simulink model "v01_aa_rii_16r4t11f_ver122sa"  (Simulink 7.6)
settings: Solver=ode45  StartTime=0.0  StopTime=128000  RelTol=1e-3
undefined symbols (not defined in the file or in its folder): sfp
libraries: xbsIndex_r4 x27287, monroe_library x154, xbsReplacements_r4 x78, casper_library_misc x33, simulink x32, dspsigops x32, xps_library x24
(part 33 of 178)

== system v01_aa_rii_16r4t11f_ver122sa/pfb_fir_real1/coeff_gen_6_9/cram_upper ==
  in0 : Inport
  in1 : Inport  Port=2
  in2 : Inport  Port=3
  in3 : Inport  Port=4
  concat : xbsIndex_r4/Concat  num_inputs=4  dbl_ovrd=off  block_type=concat
  reinterpret_{1..4} (4 alike) : xbsIndex_r4/Reinterpret  force_arith_type=on  arith_type=Unsigned  force_bin_pt=on  bin_pt=0  block_type=reinterpret
  out : Outport
  wires:
  concat/1 -> out/1
  reinterpret_4/1 -> concat/4
  reinterpret_3/1 -> concat/3
  reinterpret_2/1 -> concat/2
  reinterpret_1/1 -> concat/1
  in3/1 -> reinterpret_4/1
  in2/1 -> reinterpret_3/1
  in1/1 -> reinterpret_2/1
  in0/1 -> reinterpret_1/1

== system v01_aa_rii_16r4t11f_ver122sa/pfb_fir_real1/coeff_gen_7_8/cram_lower ==
  in0 : Inport
  in1 : Inport  Port=2
  in2 : Inport  Port=3
  in3 : Inport  Port=4
  concat : xbsIndex_r4/Concat  num_inputs=4  dbl_ovrd=off  block_type=concat
  reinterpret_{1..4} (4 alike) : xbsIndex_r4/Reinterpret  force_arith_type=on  arith_type=Unsigned  force_bin_pt=on  bin_pt=0  block_type=reinterpret
  out : Outport
  wires:
  concat/1 -> out/1
  reinterpret_4/1 -> concat/4
  reinterpret_3/1 -> concat/3
  reinterpret_2/1 -> concat/2
  reinterpret_1/1 -> concat/1
  in3/1 -> reinterpret_4/1
  in2/1 -> reinterpret_3/1
  in1/1 -> reinterpret_2/1
  in0/1 -> reinterpret_1/1

== system v01_aa_rii_16r4t11f_ver122sa/pfb_fir_real1/coeff_gen_7_8/cram_upper ==
  in0 : Inport
  in1 : Inport  Port=2
  in2 : Inport  Port=3
  in3 : Inport  Port=4
  concat : xbsIndex_r4/Concat  num_inputs=4  dbl_ovrd=off  block_type=concat
  reinterpret_{1..4} (4 alike) : xbsIndex_r4/Reinterpret  force_arith_type=on  arith_type=Unsigned  force_bin_pt=on  bin_pt=0  block_type=reinterpret
  out : Outport
  wires:
  concat/1 -> out/1
  reinterpret_4/1 -> concat/4
  reinterpret_3/1 -> concat/3
  reinterpret_2/1 -> concat/2
  reinterpret_1/1 -> concat/1
  in3/1 -> reinterpret_4/1
  in2/1 -> reinterpret_3/1
  in1/1 -> reinterpret_2/1
  in0/1 -> reinterpret_1/1

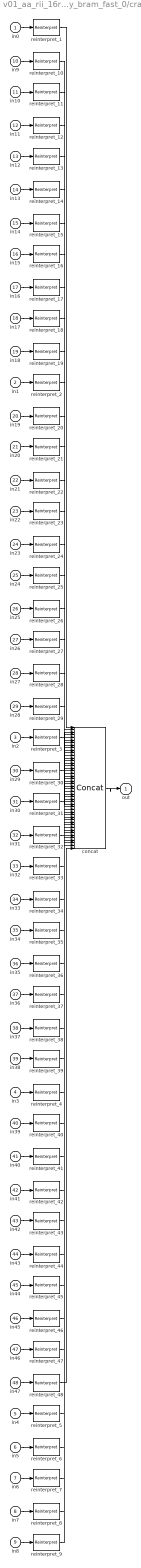
== system v01_aa_rii_16r4t11f_ver122sa/pfb_fir_real1/multi_delay_bram_fast_0/cram ==
  in0 : Inport
  in1 : Inport  Port=2
  in2 : Inport  Port=3
  in3 : Inport  Port=4
  in4 : Inport  Port=5
  in5 : Inport  Port=6
  in6 : Inport  Port=7
  in7 : Inport  Port=8
  in8 : Inport  Port=9
  in9 : Inport  Port=10
  in10 : Inport  Port=11
  in11 : Inport  Port=12
  in12 : Inport  Port=13
  in13 : Inport  Port=14
  in14 : Inport  Port=15
  in15 : Inport  Port=16
  in16 : Inport  Port=17
  in17 : Inport  Port=18
  in18 : Inport  Port=19
  in19 : Inport  Port=20
  in20 : Inport  Port=21
  in21 : Inport  Port=22
  in22 : Inport  Port=23
  in23 : Inport  Port=24
  in24 : Inport  Port=25
  in25 : Inport  Port=26
  in26 : Inport  Port=27
  in27 : Inport  Port=28
  in28 : Inport  Port=29
  in29 : Inport  Port=30
  in30 : Inport  Port=31
  in31 : Inport  Port=32
  in32 : Inport  Port=33
  in33 : Inport  Port=34
  in34 : Inport  Port=35
  in35 : Inport  Port=36
  in36 : Inport  Port=37
  in37 : Inport  Port=38
  in38 : Inport  Port=39
  in39 : Inport  Port=40
  in40 : Inport  Port=41
  in41 : Inport  Port=42
  in42 : Inport  Port=43
  in43 : Inport  Port=44
  in44 : Inport  Port=45
  in45 : Inport  Port=46
  in46 : Inport  Port=47
  in47 : Inport  Port=48
  concat : xbsIndex_r4/Concat  num_inputs=48  dbl_ovrd=off  block_type=concat
  reinterpret_{1..48} (48 alike) : xbsIndex_r4/Reinterpret  force_arith_type=on  arith_type=Unsigned  force_bin_pt=on  bin_pt=0  block_type=reinterpret
  out : Outport
  wires:
  concat/1 -> out/1
  reinterpret_48/1 -> concat/48
  reinterpret_47/1 -> concat/47
  reinterpret_46/1 -> concat/46
  reinterpret_45/1 -> concat/45
  reinterpret_44/1 -> concat/44
  reinterpret_43/1 -> concat/43
  reinterpret_42/1 -> concat/42
  reinterpret_41/1 -> concat/41
  reinterpret_40/1 -> concat/40
  reinterpret_39/1 -> concat/39
  reinterpret_38/1 -> concat/38
  reinterpret_37/1 -> concat/37
  reinterpret_36/1 -> concat/36
  reinterpret_35/1 -> concat/35
  reinterpret_34/1 -> concat/34
  reinterpret_33/1 -> concat/33
  reinterpret_32/1 -> concat/32
  reinterpret_31/1 -> concat/31
  reinterpret_30/1 -> concat/30
  reinterpret_29/1 -> concat/29
  reinterpret_28/1 -> concat/28
  reinterpret_27/1 -> concat/27
  reinterpret_26/1 -> concat/26
  reinterpret_25/1 -> concat/25
  reinterpret_24/1 -> concat/24
  reinterpret_23/1 -> concat/23
  reinterpret_22/1 -> concat/22
  reinterpret_21/1 -> concat/21
  reinterpret_20/1 -> concat/20
  reinterpret_19/1 -> concat/19
  reinterpret_18/1 -> concat/18
  reinterpret_17/1 -> concat/17
  reinterpret_16/1 -> concat/16
  reinterpret_15/1 -> concat/15
  reinterpret_14/1 -> concat/14
  reinterpret_13/1 -> concat/13
  reinterpret_12/1 -> concat/12
  reinterpret_11/1 -> concat/11
  reinterpret_10/1 -> concat/10
  reinterpret_9/1 -> concat/9
  reinterpret_8/1 -> concat/8
  reinterpret_7/1 -> concat/7
  reinterpret_6/1 -> concat/6
  reinterpret_5/1 -> concat/5
  reinterpret_4/1 -> concat/4
  reinterpret_3/1 -> concat/3
  reinterpret_2/1 -> concat/2
  reinterpret_1/1 -> concat/1
  in47/1 -> reinterpret_48/1
  in46/1 -> reinterpret_47/1
  in45/1 -> reinterpret_46/1
  in44/1 -> reinterpret_45/1
  in43/1 -> reinterpret_44/1
  in42/1 -> reinterpret_43/1
  in41/1 -> reinterpret_42/1
  in40/1 -> reinterpret_41/1
  in39/1 -> reinterpret_40/1
  in38/1 -> reinterpret_39/1
  in37/1 -> reinterpret_38/1
  in36/1 -> reinterpret_37/1
  in35/1 -> reinterpret_36/1
  in34/1 -> reinterpret_35/1
  in33/1 -> reinterpret_34/1
  in32/1 -> reinterpret_33/1
  in31/1 -> reinterpret_32/1
  in30/1 -> reinterpret_31/1
  in29/1 -> reinterpret_30/1
  in28/1 -> reinterpret_29/1
  in27/1 -> reinterpret_28/1
  in26/1 -> reinterpret_27/1
  in25/1 -> reinterpret_26/1
  in24/1 -> reinterpret_25/1
  in23/1 -> reinterpret_24/1
  in22/1 -> reinterpret_23/1
  in21/1 -> reinterpret_22/1
  in20/1 -> reinterpret_21/1
  in19/1 -> reinterpret_20/1
  in18/1 -> reinterpret_19/1
  in17/1 -> reinterpret_18/1
  in16/1 -> reinterpret_17/1
  in15/1 -> reinterpret_16/1
  in14/1 -> reinterpret_15/1
  in13/1 -> reinterpret_14/1
  in12/1 -> reinterpret_13/1
  in11/1 -> reinterpret_12/1
  in10/1 -> reinterpret_11/1
  in9/1 -> reinterpret_10/1
  in8/1 -> reinterpret_9/1
  in7/1 -> reinterpret_8/1
  in6/1 -> reinterpret_7/1
  in5/1 -> reinterpret_6/1
  in4/1 -> reinterpret_5/1
  in3/1 -> reinterpret_4/1
  in2/1 -> reinterpret_3/1
  in1/1 -> reinterpret_2/1
  in0/1 -> reinterpret_1/1

== system v01_aa_rii_16r4t11f_ver122sa/pfb_fir_real1/multi_delay_bram_fast_0/cram_all ==
  in0 : Inport
  in1 : Inport  Port=2
  in2 : Inport  Port=3
  in3 : Inport  Port=4
  in4 : Inport  Port=5
  in5 : Inport  Port=6
  in6 : Inport  Port=7
  in7 : Inport  Port=8
  in8 : Inport  Port=9
  in9 : Inport  Port=10
  in10 : Inport  Port=11
  concat : xbsIndex_r4/Concat  num_inputs=11  dbl_ovrd=off  block_type=concat
  reinterpret_{1..11} (11 alike) : xbsIndex_r4/Reinterpret  force_arith_type=on  arith_type=Unsigned  force_bin_pt=on  bin_pt=0  block_type=reinterpret
  out : Outport
  wires:
  concat/1 -> out/1
  reinterpret_11/1 -> concat/11
  reinterpret_10/1 -> concat/10
  reinterpret_9/1 -> concat/9
  reinterpret_8/1 -> concat/8
  reinterpret_7/1 -> concat/7
  reinterpret_6/1 -> concat/6
  reinterpret_5/1 -> concat/5
  reinterpret_4/1 -> concat/4
  reinterpret_3/1 -> concat/3
  reinterpret_2/1 -> concat/2
  reinterpret_1/1 -> concat/1
  in10/1 -> reinterpret_11/1
  in9/1 -> reinterpret_10/1
  in8/1 -> reinterpret_9/1
  in7/1 -> reinterpret_8/1
  in6/1 -> reinterpret_7/1
  in5/1 -> reinterpret_6/1
  in4/1 -> reinterpret_5/1
  in3/1 -> reinterpret_4/1
  in2/1 -> reinterpret_3/1
  in1/1 -> reinterpret_2/1
  in0/1 -> reinterpret_1/1

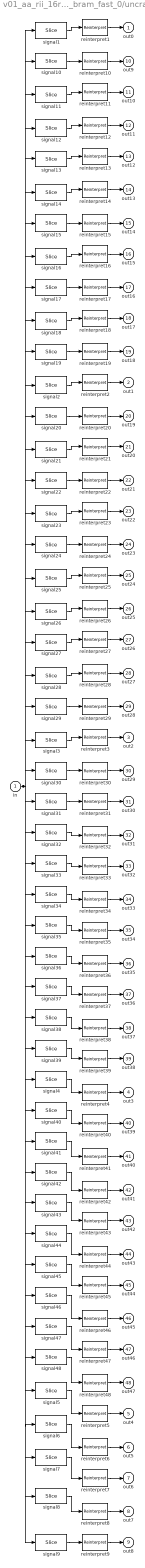
== system v01_aa_rii_16r4t11f_ver122sa/pfb_fir_real1/multi_delay_bram_fast_0/uncram ==
  in : Inport
  reinterpret{1..48} (48 alike) : xbsIndex_r4/Reinterpret  force_arith_type=on  arith_type="Signed  (2's comp)"  force_bin_pt=on  bin_pt=7  block_type=reinterpret
  signal1 : xbsIndex_r4/Slice  nbits=8  boolean_output=off  mode=Upper Bit Location + Width  bit1=-0  base1=MSB of Input  bit0=-0  base0=MSB of Input  dbl_ovrd=off  block_type=slice
  signal10 : xbsIndex_r4/Slice  nbits=8  boolean_output=off  mode=Upper Bit Location + Width  bit1=-72  base1=MSB of Input  bit0=-72  base0=MSB of Input  dbl_ovrd=off  block_type=slice
  signal11 : xbsIndex_r4/Slice  nbits=8  boolean_output=off  mode=Upper Bit Location + Width  bit1=-80  base1=MSB of Input  bit0=-80  base0=MSB of Input  dbl_ovrd=off  block_type=slice
  signal12 : xbsIndex_r4/Slice  nbits=8  boolean_output=off  mode=Upper Bit Location + Width  bit1=-88  base1=MSB of Input  bit0=-88  base0=MSB of Input  dbl_ovrd=off  block_type=slice
  signal13 : xbsIndex_r4/Slice  nbits=8  boolean_output=off  mode=Upper Bit Location + Width  bit1=-96  base1=MSB of Input  bit0=-96  base0=MSB of Input  dbl_ovrd=off  block_type=slice
  signal14 : xbsIndex_r4/Slice  nbits=8  boolean_output=off  mode=Upper Bit Location + Width  bit1=-104  base1=MSB of Input  bit0=-104  base0=MSB of Input  dbl_ovrd=off  block_type=slice
  signal15 : xbsIndex_r4/Slice  nbits=8  boolean_output=off  mode=Upper Bit Location + Width  bit1=-112  base1=MSB of Input  bit0=-112  base0=MSB of Input  dbl_ovrd=off  block_type=slice
  signal16 : xbsIndex_r4/Slice  nbits=8  boolean_output=off  mode=Upper Bit Location + Width  bit1=-120  base1=MSB of Input  bit0=-120  base0=MSB of Input  dbl_ovrd=off  block_type=slice
  signal17 : xbsIndex_r4/Slice  nbits=8  boolean_output=off  mode=Upper Bit Location + Width  bit1=-128  base1=MSB of Input  bit0=-128  base0=MSB of Input  dbl_ovrd=off  block_type=slice
  signal18 : xbsIndex_r4/Slice  nbits=8  boolean_output=off  mode=Upper Bit Location + Width  bit1=-136  base1=MSB of Input  bit0=-136  base0=MSB of Input  dbl_ovrd=off  block_type=slice
  signal19 : xbsIndex_r4/Slice  nbits=8  boolean_output=off  mode=Upper Bit Location + Width  bit1=-144  base1=MSB of Input  bit0=-144  base0=MSB of Input  dbl_ovrd=off  block_type=slice
  signal2 : xbsIndex_r4/Slice  nbits=8  boolean_output=off  mode=Upper Bit Location + Width  bit1=-8  base1=MSB of Input  bit0=-8  base0=MSB of Input  dbl_ovrd=off  block_type=slice
  signal20 : xbsIndex_r4/Slice  nbits=8  boolean_output=off  mode=Upper Bit Location + Width  bit1=-152  base1=MSB of Input  bit0=-152  base0=MSB of Input  dbl_ovrd=off  block_type=slice
  signal21 : xbsIndex_r4/Slice  nbits=8  boolean_output=off  mode=Upper Bit Location + Width  bit1=-160  base1=MSB of Input  bit0=-160  base0=MSB of Input  dbl_ovrd=off  block_type=slice
  signal22 : xbsIndex_r4/Slice  nbits=8  boolean_output=off  mode=Upper Bit Location + Width  bit1=-168  base1=MSB of Input  bit0=-168  base0=MSB of Input  dbl_ovrd=off  block_type=slice
  signal23 : xbsIndex_r4/Slice  nbits=8  boolean_output=off  mode=Upper Bit Location + Width  bit1=-176  base1=MSB of Input  bit0=-176  base0=MSB of Input  dbl_ovrd=off  block_type=slice
  signal24 : xbsIndex_r4/Slice  nbits=8  boolean_output=off  mode=Upper Bit Location + Width  bit1=-184  base1=MSB of Input  bit0=-184  base0=MSB of Input  dbl_ovrd=off  block_type=slice
  signal25 : xbsIndex_r4/Slice  nbits=8  boolean_output=off  mode=Upper Bit Location + Width  bit1=-192  base1=MSB of Input  bit0=-192  base0=MSB of Input  dbl_ovrd=off  block_type=slice
  signal26 : xbsIndex_r4/Slice  nbits=8  boolean_output=off  mode=Upper Bit Location + Width  bit1=-200  base1=MSB of Input  bit0=-200  base0=MSB of Input  dbl_ovrd=off  block_type=slice
  signal27 : xbsIndex_r4/Slice  nbits=8  boolean_output=off  mode=Upper Bit Location + Width  bit1=-208  base1=MSB of Input  bit0=-208  base0=MSB of Input  dbl_ovrd=off  block_type=slice
  signal28 : xbsIndex_r4/Slice  nbits=8  boolean_output=off  mode=Upper Bit Location + Width  bit1=-216  base1=MSB of Input  bit0=-216  base0=MSB of Input  dbl_ovrd=off  block_type=slice
  signal29 : xbsIndex_r4/Slice  nbits=8  boolean_output=off  mode=Upper Bit Location + Width  bit1=-224  base1=MSB of Input  bit0=-224  base0=MSB of Input  dbl_ovrd=off  block_type=slice
  signal3 : xbsIndex_r4/Slice  nbits=8  boolean_output=off  mode=Upper Bit Location + Width  bit1=-16  base1=MSB of Input  bit0=-16  base0=MSB of Input  dbl_ovrd=off  block_type=slice
  signal30 : xbsIndex_r4/Slice  nbits=8  boolean_output=off  mode=Upper Bit Location + Width  bit1=-232  base1=MSB of Input  bit0=-232  base0=MSB of Input  dbl_ovrd=off  block_type=slice
  signal31 : xbsIndex_r4/Slice  nbits=8  boolean_output=off  mode=Upper Bit Location + Width  bit1=-240  base1=MSB of Input  bit0=-240  base0=MSB of Input  dbl_ovrd=off  block_type=slice
  signal32 : xbsIndex_r4/Slice  nbits=8  boolean_output=off  mode=Upper Bit Location + Width  bit1=-248  base1=MSB of Input  bit0=-248  base0=MSB of Input  dbl_ovrd=off  block_type=slice
  signal33 : xbsIndex_r4/Slice  nbits=8  boolean_output=off  mode=Upper Bit Location + Width  bit1=-256  base1=MSB of Input  bit0=-256  base0=MSB of Input  dbl_ovrd=off  block_type=slice
  signal34 : xbsIndex_r4/Slice  nbits=8  boolean_output=off  mode=Upper Bit Location + Width  bit1=-264  base1=MSB of Input  bit0=-264  base0=MSB of Input  dbl_ovrd=off  block_type=slice
  signal35 : xbsIndex_r4/Slice  nbits=8  boolean_output=off  mode=Upper Bit Location + Width  bit1=-272  base1=MSB of Input  bit0=-272  base0=MSB of Input  dbl_ovrd=off  block_type=slice
  signal36 : xbsIndex_r4/Slice  nbits=8  boolean_output=off  mode=Upper Bit Location + Width  bit1=-280  base1=MSB of Input  bit0=-280  base0=MSB of Input  dbl_ovrd=off  block_type=slice
  signal37 : xbsIndex_r4/Slice  nbits=8  boolean_output=off  mode=Upper Bit Location + Width  bit1=-288  base1=MSB of Input  bit0=-288  base0=MSB of Input  dbl_ovrd=off  block_type=slice
  signal38 : xbsIndex_r4/Slice  nbits=8  boolean_output=off  mode=Upper Bit Location + Width  bit1=-296  base1=MSB of Input  bit0=-296  base0=MSB of Input  dbl_ovrd=off  block_type=slice
  signal39 : xbsIndex_r4/Slice  nbits=8  boolean_output=off  mode=Upper Bit Location + Width  bit1=-304  base1=MSB of Input  bit0=-304  base0=MSB of Input  dbl_ovrd=off  block_type=slice
  signal4 : xbsIndex_r4/Slice  nbits=8  boolean_output=off  mode=Upper Bit Location + Width  bit1=-24  base1=MSB of Input  bit0=-24  base0=MSB of Input  dbl_ovrd=off  block_type=slice
  signal40 : xbsIndex_r4/Slice  nbits=8  boolean_output=off  mode=Upper Bit Location + Width  bit1=-312  base1=MSB of Input  bit0=-312  base0=MSB of Input  dbl_ovrd=off  block_type=slice
  signal41 : xbsIndex_r4/Slice  nbits=8  boolean_output=off  mode=Upper Bit Location + Width  bit1=-320  base1=MSB of Input  bit0=-320  base0=MSB of Input  dbl_ovrd=off  block_type=slice
  signal42 : xbsIndex_r4/Slice  nbits=8  boolean_output=off  mode=Upper Bit Location + Width  bit1=-328  base1=MSB of Input  bit0=-328  base0=MSB of Input  dbl_ovrd=off  block_type=slice
  signal43 : xbsIndex_r4/Slice  nbits=8  boolean_output=off  mode=Upper Bit Location + Width  bit1=-336  base1=MSB of Input  bit0=-336  base0=MSB of Input  dbl_ovrd=off  block_type=slice
  signal44 : xbsIndex_r4/Slice  nbits=8  boolean_output=off  mode=Upper Bit Location + Width  bit1=-344  base1=MSB of Input  bit0=-344  base0=MSB of Input  dbl_ovrd=off  block_type=slice
  signal45 : xbsIndex_r4/Slice  nbits=8  boolean_output=off  mode=Upper Bit Location + Width  bit1=-352  base1=MSB of Input  bit0=-352  base0=MSB of Input  dbl_ovrd=off  block_type=slice
  signal46 : xbsIndex_r4/Slice  nbits=8  boolean_output=off  mode=Upper Bit Location + Width  bit1=-360  base1=MSB of Input  bit0=-360  base0=MSB of Input  dbl_ovrd=off  block_type=slice
  signal47 : xbsIndex_r4/Slice  nbits=8  boolean_output=off  mode=Upper Bit Location + Width  bit1=-368  base1=MSB of Input  bit0=-368  base0=MSB of Input  dbl_ovrd=off  block_type=slice
  signal48 : xbsIndex_r4/Slice  nbits=8  boolean_output=off  mode=Upper Bit Location + Width  bit1=-376  base1=MSB of Input  bit0=-376  base0=MSB of Input  dbl_ovrd=off  block_type=slice
  signal5 : xbsIndex_r4/Slice  nbits=8  boolean_output=off  mode=Upper Bit Location + Width  bit1=-32  base1=MSB of Input  bit0=-32  base0=MSB of Input  dbl_ovrd=off  block_type=slice
  signal6 : xbsIndex_r4/Slice  nbits=8  boolean_output=off  mode=Upper Bit Location + Width  bit1=-40  base1=MSB of Input  bit0=-40  base0=MSB of Input  dbl_ovrd=off  block_type=slice
  signal7 : xbsIndex_r4/Slice  nbits=8  boolean_output=off  mode=Upper Bit Location + Width  bit1=-48  base1=MSB of Input  bit0=-48  base0=MSB of Input  dbl_ovrd=off  block_type=slice
  signal8 : xbsIndex_r4/Slice  nbits=8  boolean_output=off  mode=Upper Bit Location + Width  bit1=-56  base1=MSB of Input  bit0=-56  base0=MSB of Input  dbl_ovrd=off  block_type=slice
  signal9 : xbsIndex_r4/Slice  nbits=8  boolean_output=off  mode=Upper Bit Location + Width  bit1=-64  base1=MSB of Input  bit0=-64  base0=MSB of Input  dbl_ovrd=off  block_type=slice
  out0 : Outport
  out1 : Outport  Port=2
  out2 : Outport  Port=3
  out3 : Outport  Port=4
  out4 : Outport  Port=5
  out5 : Outport  Port=6
  out6 : Outport  Port=7
  out7 : Outport  Port=8
  out8 : Outport  Port=9
  out9 : Outport  Port=10
  out10 : Outport  Port=11
  out11 : Outport  Port=12
  out12 : Outport  Port=13
  out13 : Outport  Port=14
  out14 : Outport  Port=15
  out15 : Outport  Port=16
  out16 : Outport  Port=17
  out17 : Outport  Port=18
  out18 : Outport  Port=19
  out19 : Outport  Port=20
  out20 : Outport  Port=21
  out21 : Outport  Port=22
  out22 : Outport  Port=23
  out23 : Outport  Port=24
  out24 : Outport  Port=25
  out25 : Outport  Port=26
  out26 : Outport  Port=27
  out27 : Outport  Port=28
  out28 : Outport  Port=29
  out29 : Outport  Port=30
  out30 : Outport  Port=31
  out31 : Outport  Port=32
  out32 : Outport  Port=33
  out33 : Outport  Port=34
  out34 : Outport  Port=35
  out35 : Outport  Port=36
  out36 : Outport  Port=37
  out37 : Outport  Port=38
  out38 : Outport  Port=39
  out39 : Outport  Port=40
  out40 : Outport  Port=41
  out41 : Outport  Port=42
  out42 : Outport  Port=43
  out43 : Outport  Port=44
  out44 : Outport  Port=45
  out45 : Outport  Port=46
  out46 : Outport  Port=47
  out47 : Outport  Port=48
  wires:
  in/1 -> signal48/1, signal47/1, signal46/1, signal45/1, signal44/1, signal43/1, signal42/1, signal41/1, signal40/1, signal39/1, signal38/1, signal37/1, signal36/1, signal35/1, signal34/1, signal33/1, signal32/1, signal31/1, signal30/1, signal29/1, signal28/1, signal27/1, signal26/1, signal25/1, signal24/1, signal23/1, signal22/1, signal21/1, signal20/1, signal19/1, signal18/1, signal17/1, signal16/1, signal15/1, signal14/1, signal13/1, signal12/1, signal11/1, signal10/1, signal9/1, signal8/1, signal7/1, signal6/1, signal5/1, signal4/1, signal3/1, signal2/1, signal1/1
  reinterpret48/1 -> out47/1
  signal48/1 -> reinterpret48/1
  reinterpret47/1 -> out46/1
  signal47/1 -> reinterpret47/1
  reinterpret46/1 -> out45/1
  signal46/1 -> reinterpret46/1
  reinterpret45/1 -> out44/1
  signal45/1 -> reinterpret45/1
  reinterpret44/1 -> out43/1
  signal44/1 -> reinterpret44/1
  reinterpret43/1 -> out42/1
  signal43/1 -> reinterpret43/1
  reinterpret42/1 -> out41/1
  signal42/1 -> reinterpret42/1
  reinterpret41/1 -> out40/1
  signal41/1 -> reinterpret41/1
  reinterpret40/1 -> out39/1
  signal40/1 -> reinterpret40/1
  reinterpret39/1 -> out38/1
  signal39/1 -> reinterpret39/1
  reinterpret38/1 -> out37/1
  signal38/1 -> reinterpret38/1
  reinterpret37/1 -> out36/1
  signal37/1 -> reinterpret37/1
  reinterpret36/1 -> out35/1
  signal36/1 -> reinterpret36/1
  reinterpret35/1 -> out34/1
  signal35/1 -> reinterpret35/1
  reinterpret34/1 -> out33/1
  signal34/1 -> reinterpret34/1
  reinterpret33/1 -> out32/1
  signal33/1 -> reinterpret33/1
  reinterpret32/1 -> out31/1
  signal32/1 -> reinterpret32/1
  reinterpret31/1 -> out30/1
  signal31/1 -> reinterpret31/1
  reinterpret30/1 -> out29/1
  signal30/1 -> reinterpret30/1
  reinterpret29/1 -> out28/1
  signal29/1 -> reinterpret29/1
  reinterpret28/1 -> out27/1
  signal28/1 -> reinterpret28/1
  reinterpret27/1 -> out26/1
  signal27/1 -> reinterpret27/1
  reinterpret26/1 -> out25/1
  signal26/1 -> reinterpret26/1
  reinterpret25/1 -> out24/1
  signal25/1 -> reinterpret25/1
  reinterpret24/1 -> out23/1
  signal24/1 -> reinterpret24/1
  reinterpret23/1 -> out22/1
  signal23/1 -> reinterpret23/1
  reinterpret22/1 -> out21/1
  signal22/1 -> reinterpret22/1
  reinterpret21/1 -> out20/1
  signal21/1 -> reinterpret21/1
  reinterpret20/1 -> out19/1
  signal20/1 -> reinterpret20/1
  reinterpret19/1 -> out18/1
  signal19/1 -> reinterpret19/1
  reinterpret18/1 -> out17/1
  signal18/1 -> reinterpret18/1
  reinterpret17/1 -> out16/1
  signal17/1 -> reinterpret17/1
  reinterpret16/1 -> out15/1
  signal16/1 -> reinterpret16/1
  reinterpret15/1 -> out14/1
  signal15/1 -> reinterpret15/1
  reinterpret14/1 -> out13/1
  signal14/1 -> reinterpret14/1
  reinterpret13/1 -> out12/1
  signal13/1 -> reinterpret13/1
  reinterpret12/1 -> out11/1
  signal12/1 -> reinterpret12/1
  reinterpret11/1 -> out10/1
  signal11/1 -> reinterpret11/1
  reinterpret10/1 -> out9/1
  signal10/1 -> reinterpret10/1
  reinterpret9/1 -> out8/1
  signal9/1 -> reinterpret9/1
  reinterpret8/1 -> out7/1
  signal8/1 -> reinterpret8/1
  reinterpret7/1 -> out6/1
  signal7/1 -> reinterpret7/1
  reinterpret6/1 -> out5/1
  signal6/1 -> reinterpret6/1
  reinterpret5/1 -> out4/1
  signal5/1 -> reinterpret5/1
  reinterpret4/1 -> out3/1
  signal4/1 -> reinterpret4/1
  reinterpret3/1 -> out2/1
  signal3/1 -> reinterpret3/1
  reinterpret2/1 -> out1/1
  signal2/1 -> reinterpret2/1
  reinterpret1/1 -> out0/1
  signal1/1 -> reinterpret1/1

== system v01_aa_rii_16r4t11f_ver122sa/pfb_fir_real2/coeff_gen_0_15/cram_lower ==
  in0 : Inport
  in1 : Inport  Port=2
  in2 : Inport  Port=3
  in3 : Inport  Port=4
  concat : xbsIndex_r4/Concat  num_inputs=4  dbl_ovrd=off  block_type=concat
  reinterpret_{1..4} (4 alike) : xbsIndex_r4/Reinterpret  force_arith_type=on  arith_type=Unsigned  force_bin_pt=on  bin_pt=0  block_type=reinterpret
  out : Outport
  wires:
  concat/1 -> out/1
  reinterpret_4/1 -> concat/4
  reinterpret_3/1 -> concat/3
  reinterpret_2/1 -> concat/2
  reinterpret_1/1 -> concat/1
  in3/1 -> reinterpret_4/1
  in2/1 -> reinterpret_3/1
  in1/1 -> reinterpret_2/1
  in0/1 -> reinterpret_1/1

== system v01_aa_rii_16r4t11f_ver122sa/pfb_fir_real2/coeff_gen_0_15/cram_upper ==
  in0 : Inport
  in1 : Inport  Port=2
  in2 : Inport  Port=3
  in3 : Inport  Port=4
  concat : xbsIndex_r4/Concat  num_inputs=4  dbl_ovrd=off  block_type=concat
  reinterpret_{1..4} (4 alike) : xbsIndex_r4/Reinterpret  force_arith_type=on  arith_type=Unsigned  force_bin_pt=on  bin_pt=0  block_type=reinterpret
  out : Outport
  wires:
  concat/1 -> out/1
  reinterpret_4/1 -> concat/4
  reinterpret_3/1 -> concat/3
  reinterpret_2/1 -> concat/2
  reinterpret_1/1 -> concat/1
  in3/1 -> reinterpret_4/1
  in2/1 -> reinterpret_3/1
  in1/1 -> reinterpret_2/1
  in0/1 -> reinterpret_1/1

== system v01_aa_rii_16r4t11f_ver122sa/pfb_fir_real2/coeff_gen_1_14/cram_lower ==
  in0 : Inport
  in1 : Inport  Port=2
  in2 : Inport  Port=3
  in3 : Inport  Port=4
  concat : xbsIndex_r4/Concat  num_inputs=4  dbl_ovrd=off  block_type=concat
  reinterpret_{1..4} (4 alike) : xbsIndex_r4/Reinterpret  force_arith_type=on  arith_type=Unsigned  force_bin_pt=on  bin_pt=0  block_type=reinterpret
  out : Outport
  wires:
  concat/1 -> out/1
  reinterpret_4/1 -> concat/4
  reinterpret_3/1 -> concat/3
  reinterpret_2/1 -> concat/2
  reinterpret_1/1 -> concat/1
  in3/1 -> reinterpret_4/1
  in2/1 -> reinterpret_3/1
  in1/1 -> reinterpret_2/1
  in0/1 -> reinterpret_1/1

== system v01_aa_rii_16r4t11f_ver122sa/pfb_fir_real2/coeff_gen_1_14/cram_upper ==
  in0 : Inport
  in1 : Inport  Port=2
  in2 : Inport  Port=3
  in3 : Inport  Port=4
  concat : xbsIndex_r4/Concat  num_inputs=4  dbl_ovrd=off  block_type=concat
  reinterpret_{1..4} (4 alike) : xbsIndex_r4/Reinterpret  force_arith_type=on  arith_type=Unsigned  force_bin_pt=on  bin_pt=0  block_type=reinterpret
  out : Outport
  wires:
  concat/1 -> out/1
  reinterpret_4/1 -> concat/4
  reinterpret_3/1 -> concat/3
  reinterpret_2/1 -> concat/2
  reinterpret_1/1 -> concat/1
  in3/1 -> reinterpret_4/1
  in2/1 -> reinterpret_3/1
  in1/1 -> reinterpret_2/1
  in0/1 -> reinterpret_1/1

== system v01_aa_rii_16r4t11f_ver122sa/pfb_fir_real2/coeff_gen_2_13/cram_lower ==
  in0 : Inport
  in1 : Inport  Port=2
  in2 : Inport  Port=3
  in3 : Inport  Port=4
  concat : xbsIndex_r4/Concat  num_inputs=4  dbl_ovrd=off  block_type=concat
  reinterpret_{1..4} (4 alike) : xbsIndex_r4/Reinterpret  force_arith_type=on  arith_type=Unsigned  force_bin_pt=on  bin_pt=0  block_type=reinterpret
  out : Outport
  wires:
  concat/1 -> out/1
  reinterpret_4/1 -> concat/4
  reinterpret_3/1 -> concat/3
  reinterpret_2/1 -> concat/2
  reinterpret_1/1 -> concat/1
  in3/1 -> reinterpret_4/1
  in2/1 -> reinterpret_3/1
  in1/1 -> reinterpret_2/1
  in0/1 -> reinterpret_1/1

== system v01_aa_rii_16r4t11f_ver122sa/pfb_fir_real2/coeff_gen_2_13/cram_upper ==
  in0 : Inport
  in1 : Inport  Port=2
  in2 : Inport  Port=3
  in3 : Inport  Port=4
  concat : xbsIndex_r4/Concat  num_inputs=4  dbl_ovrd=off  block_type=concat
  reinterpret_{1..4} (4 alike) : xbsIndex_r4/Reinterpret  force_arith_type=on  arith_type=Unsigned  force_bin_pt=on  bin_pt=0  block_type=reinterpret
  out : Outport
  wires:
  concat/1 -> out/1
  reinterpret_4/1 -> concat/4
  reinterpret_3/1 -> concat/3
  reinterpret_2/1 -> concat/2
  reinterpret_1/1 -> concat/1
  in3/1 -> reinterpret_4/1
  in2/1 -> reinterpret_3/1
  in1/1 -> reinterpret_2/1
  in0/1 -> reinterpret_1/1

== system v01_aa_rii_16r4t11f_ver122sa/pfb_fir_real2/coeff_gen_3_12/cram_lower ==
  in0 : Inport
  in1 : Inport  Port=2
  in2 : Inport  Port=3
  in3 : Inport  Port=4
  concat : xbsIndex_r4/Concat  num_inputs=4  dbl_ovrd=off  block_type=concat
  reinterpret_{1..4} (4 alike) : xbsIndex_r4/Reinterpret  force_arith_type=on  arith_type=Unsigned  force_bin_pt=on  bin_pt=0  block_type=reinterpret
  out : Outport
  wires:
  concat/1 -> out/1
  reinterpret_4/1 -> concat/4
  reinterpret_3/1 -> concat/3
  reinterpret_2/1 -> concat/2
  reinterpret_1/1 -> concat/1
  in3/1 -> reinterpret_4/1
  in2/1 -> reinterpret_3/1
  in1/1 -> reinterpret_2/1
  in0/1 -> reinterpret_1/1

== system v01_aa_rii_16r4t11f_ver122sa/pfb_fir_real2/coeff_gen_3_12/cram_upper ==
  in0 : Inport
  in1 : Inport  Port=2
  in2 : Inport  Port=3
  in3 : Inport  Port=4
  concat : xbsIndex_r4/Concat  num_inputs=4  dbl_ovrd=off  block_type=concat
  reinterpret_{1..4} (4 alike) : xbsIndex_r4/Reinterpret  force_arith_type=on  arith_type=Unsigned  force_bin_pt=on  bin_pt=0  block_type=reinterpret
  out : Outport
  wires:
  concat/1 -> out/1
  reinterpret_4/1 -> concat/4
  reinterpret_3/1 -> concat/3
  reinterpret_2/1 -> concat/2
  reinterpret_1/1 -> concat/1
  in3/1 -> reinterpret_4/1
  in2/1 -> reinterpret_3/1
  in1/1 -> reinterpret_2/1
  in0/1 -> reinterpret_1/1

== system v01_aa_rii_16r4t11f_ver122sa/pfb_fir_real2/coeff_gen_4_11/cram_lower ==
  in0 : Inport
  in1 : Inport  Port=2
  in2 : Inport  Port=3
  in3 : Inport  Port=4
  concat : xbsIndex_r4/Concat  num_inputs=4  dbl_ovrd=off  block_type=concat
  reinterpret_{1..4} (4 alike) : xbsIndex_r4/Reinterpret  force_arith_type=on  arith_type=Unsigned  force_bin_pt=on  bin_pt=0  block_type=reinterpret
  out : Outport
  wires:
  concat/1 -> out/1
  reinterpret_4/1 -> concat/4
  reinterpret_3/1 -> concat/3
  reinterpret_2/1 -> concat/2
  reinterpret_1/1 -> concat/1
  in3/1 -> reinterpret_4/1
  in2/1 -> reinterpret_3/1
  in1/1 -> reinterpret_2/1
  in0/1 -> reinterpret_1/1

== system v01_aa_rii_16r4t11f_ver122sa/pfb_fir_real2/coeff_gen_4_11/cram_upper ==
  in0 : Inport
  in1 : Inport  Port=2
  in2 : Inport  Port=3
  in3 : Inport  Port=4
  concat : xbsIndex_r4/Concat  num_inputs=4  dbl_ovrd=off  block_type=concat
  reinterpret_{1..4} (4 alike) : xbsIndex_r4/Reinterpret  force_arith_type=on  arith_type=Unsigned  force_bin_pt=on  bin_pt=0  block_type=reinterpret
  out : Outport
  wires:
  concat/1 -> out/1
  reinterpret_4/1 -> concat/4
  reinterpret_3/1 -> concat/3
  reinterpret_2/1 -> concat/2
  reinterpret_1/1 -> concat/1
  in3/1 -> reinterpret_4/1
  in2/1 -> reinterpret_3/1
  in1/1 -> reinterpret_2/1
  in0/1 -> reinterpret_1/1

== system v01_aa_rii_16r4t11f_ver122sa/pfb_fir_real2/coeff_gen_5_10/cram_lower ==
  in0 : Inport
  in1 : Inport  Port=2
  in2 : Inport  Port=3
  in3 : Inport  Port=4
  concat : xbsIndex_r4/Concat  num_inputs=4  dbl_ovrd=off  block_type=concat
  reinterpret_{1..4} (4 alike) : xbsIndex_r4/Reinterpret  force_arith_type=on  arith_type=Unsigned  force_bin_pt=on  bin_pt=0  block_type=reinterpret
  out : Outport
  wires:
  concat/1 -> out/1
  reinterpret_4/1 -> concat/4
  reinterpret_3/1 -> concat/3
  reinterpret_2/1 -> concat/2
  reinterpret_1/1 -> concat/1
  in3/1 -> reinterpret_4/1
  in2/1 -> reinterpret_3/1
  in1/1 -> reinterpret_2/1
  in0/1 -> reinterpret_1/1

== system v01_aa_rii_16r4t11f_ver122sa/pfb_fir_real2/coeff_gen_5_10/cram_upper ==
  in0 : Inport
  in1 : Inport  Port=2
  in2 : Inport  Port=3
  in3 : Inport  Port=4
  concat : xbsIndex_r4/Concat  num_inputs=4  dbl_ovrd=off  block_type=concat
  reinterpret_{1..4} (4 alike) : xbsIndex_r4/Reinterpret  force_arith_type=on  arith_type=Unsigned  force_bin_pt=on  bin_pt=0  block_type=reinterpret
  out : Outport
  wires:
  concat/1 -> out/1
  reinterpret_4/1 -> concat/4
  reinterpret_3/1 -> concat/3
  reinterpret_2/1 -> concat/2
  reinterpret_1/1 -> concat/1
  in3/1 -> reinterpret_4/1
  in2/1 -> reinterpret_3/1
  in1/1 -> reinterpret_2/1
  in0/1 -> reinterpret_1/1

== system v01_aa_rii_16r4t11f_ver122sa/pfb_fir_real2/coeff_gen_6_9/cram_lower ==
  in0 : Inport
  in1 : Inport  Port=2
  in2 : Inport  Port=3
  in3 : Inport  Port=4
  concat : xbsIndex_r4/Concat  num_inputs=4  dbl_ovrd=off  block_type=concat
  reinterpret_{1..4} (4 alike) : xbsIndex_r4/Reinterpret  force_arith_type=on  arith_type=Unsigned  force_bin_pt=on  bin_pt=0  block_type=reinterpret
  out : Outport
  wires:
  concat/1 -> out/1
  reinterpret_4/1 -> concat/4
  reinterpret_3/1 -> concat/3
  reinterpret_2/1 -> concat/2
  reinterpret_1/1 -> concat/1
  in3/1 -> reinterpret_4/1
  in2/1 -> reinterpret_3/1
  in1/1 -> reinterpret_2/1
  in0/1 -> reinterpret_1/1

== system v01_aa_rii_16r4t11f_ver122sa/pfb_fir_real2/coeff_gen_6_9/cram_upper ==
  in0 : Inport
  in1 : Inport  Port=2
  in2 : Inport  Port=3
  in3 : Inport  Port=4
  concat : xbsIndex_r4/Concat  num_inputs=4  dbl_ovrd=off  block_type=concat
  reinterpret_{1..4} (4 alike) : xbsIndex_r4/Reinterpret  force_arith_type=on  arith_type=Unsigned  force_bin_pt=on  bin_pt=0  block_type=reinterpret
  out : Outport
  wires:
  concat/1 -> out/1
  reinterpret_4/1 -> concat/4
  reinterpret_3/1 -> concat/3
  reinterpret_2/1 -> concat/2
  reinterpret_1/1 -> concat/1
  in3/1 -> reinterpret_4/1
  in2/1 -> reinterpret_3/1
  in1/1 -> reinterpret_2/1
  in0/1 -> reinterpret_1/1

== system v01_aa_rii_16r4t11f_ver122sa/pfb_fir_real2/coeff_gen_7_8/cram_lower ==
  in0 : Inport
  in1 : Inport  Port=2
  in2 : Inport  Port=3
  in3 : Inport  Port=4
  concat : xbsIndex_r4/Concat  num_inputs=4  dbl_ovrd=off  block_type=concat
  reinterpret_{1..4} (4 alike) : xbsIndex_r4/Reinterpret  force_arith_type=on  arith_type=Unsigned  force_bin_pt=on  bin_pt=0  block_type=reinterpret
  out : Outport
  wires:
  concat/1 -> out/1
  reinterpret_4/1 -> concat/4
  reinterpret_3/1 -> concat/3
  reinterpret_2/1 -> concat/2
  reinterpret_1/1 -> concat/1
  in3/1 -> reinterpret_4/1
  in2/1 -> reinterpret_3/1
  in1/1 -> reinterpret_2/1
  in0/1 -> reinterpret_1/1

== system v01_aa_rii_16r4t11f_ver122sa/pfb_fir_real2/coeff_gen_7_8/cram_upper ==
  in0 : Inport
  in1 : Inport  Port=2
  in2 : Inport  Port=3
  in3 : Inport  Port=4
  concat : xbsIndex_r4/Concat  num_inputs=4  dbl_ovrd=off  block_type=concat
  reinterpret_{1..4} (4 alike) : xbsIndex_r4/Reinterpret  force_arith_type=on  arith_type=Unsigned  force_bin_pt=on  bin_pt=0  block_type=reinterpret
  out : Outport
  wires:
  concat/1 -> out/1
  reinterpret_4/1 -> concat/4
  reinterpret_3/1 -> concat/3
  reinterpret_2/1 -> concat/2
  reinterpret_1/1 -> concat/1
  in3/1 -> reinterpret_4/1
  in2/1 -> reinterpret_3/1
  in1/1 -> reinterpret_2/1
  in0/1 -> reinterpret_1/1

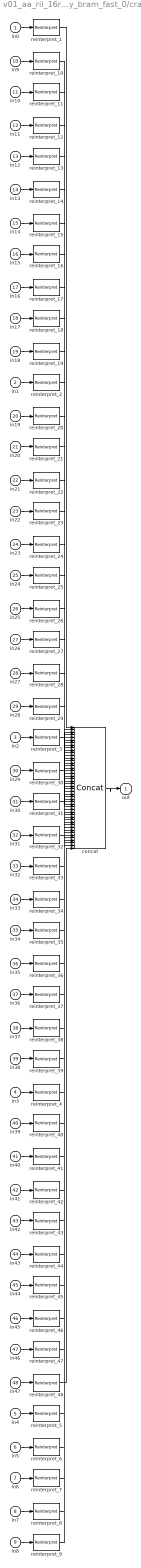
== system v01_aa_rii_16r4t11f_ver122sa/pfb_fir_real2/multi_delay_bram_fast_0/cram ==
  in0 : Inport
  in1 : Inport  Port=2
  in2 : Inport  Port=3
  in3 : Inport  Port=4
  in4 : Inport  Port=5
  in5 : Inport  Port=6
  in6 : Inport  Port=7
  in7 : Inport  Port=8
  in8 : Inport  Port=9
  in9 : Inport  Port=10
  in10 : Inport  Port=11
  in11 : Inport  Port=12
  in12 : Inport  Port=13
  in13 : Inport  Port=14
  in14 : Inport  Port=15
  in15 : Inport  Port=16
  in16 : Inport  Port=17
  in17 : Inport  Port=18
  in18 : Inport  Port=19
  in19 : Inport  Port=20
  in20 : Inport  Port=21
  in21 : Inport  Port=22
  in22 : Inport  Port=23
  in23 : Inport  Port=24
  in24 : Inport  Port=25
  in25 : Inport  Port=26
  in26 : Inport  Port=27
  in27 : Inport  Port=28
  in28 : Inport  Port=29
  in29 : Inport  Port=30
  in30 : Inport  Port=31
  in31 : Inport  Port=32
  in32 : Inport  Port=33
  in33 : Inport  Port=34
  in34 : Inport  Port=35
  in35 : Inport  Port=36
  in36 : Inport  Port=37
  in37 : Inport  Port=38
  in38 : Inport  Port=39
  in39 : Inport  Port=40
  in40 : Inport  Port=41
  in41 : Inport  Port=42
  in42 : Inport  Port=43
  in43 : Inport  Port=44
  in44 : Inport  Port=45
  in45 : Inport  Port=46
  in46 : Inport  Port=47
  in47 : Inport  Port=48
  concat : xbsIndex_r4/Concat  num_inputs=48  dbl_ovrd=off  block_type=concat
  reinterpret_{1..48} (48 alike) : xbsIndex_r4/Reinterpret  force_arith_type=on  arith_type=Unsigned  force_bin_pt=on  bin_pt=0  block_type=reinterpret
  out : Outport
  wires:
  concat/1 -> out/1
  reinterpret_48/1 -> concat/48
  reinterpret_47/1 -> concat/47
  reinterpret_46/1 -> concat/46
  reinterpret_45/1 -> concat/45
  reinterpret_44/1 -> concat/44
  reinterpret_43/1 -> concat/43
  reinterpret_42/1 -> concat/42
  reinterpret_41/1 -> concat/41
  reinterpret_40/1 -> concat/40
  reinterpret_39/1 -> concat/39
  reinterpret_38/1 -> concat/38
  reinterpret_37/1 -> concat/37
  reinterpret_36/1 -> concat/36
  reinterpret_35/1 -> concat/35
  reinterpret_34/1 -> concat/34
  reinterpret_33/1 -> concat/33
  reinterpret_32/1 -> concat/32
  reinterpret_31/1 -> concat/31
  reinterpret_30/1 -> concat/30
  reinterpret_29/1 -> concat/29
  reinterpret_28/1 -> concat/28
  reinterpret_27/1 -> concat/27
  reinterpret_26/1 -> concat/26
  reinterpret_25/1 -> concat/25
  reinterpret_24/1 -> concat/24
  reinterpret_23/1 -> concat/23
  reinterpret_22/1 -> concat/22
  reinterpret_21/1 -> concat/21
  reinterpret_20/1 -> concat/20
  reinterpret_19/1 -> concat/19
  reinterpret_18/1 -> concat/18
  reinterpret_17/1 -> concat/17
  reinterpret_16/1 -> concat/16
  reinterpret_15/1 -> concat/15
  reinterpret_14/1 -> concat/14
  reinterpret_13/1 -> concat/13
  reinterpret_12/1 -> concat/12
  reinterpret_11/1 -> concat/11
  reinterpret_10/1 -> concat/10
  reinterpret_9/1 -> concat/9
  reinterpret_8/1 -> concat/8
  reinterpret_7/1 -> concat/7
  reinterpret_6/1 -> concat/6
  reinterpret_5/1 -> concat/5
  reinterpret_4/1 -> concat/4
  reinterpret_3/1 -> concat/3
  reinterpret_2/1 -> concat/2
  reinterpret_1/1 -> concat/1
  in47/1 -> reinterpret_48/1
  in46/1 -> reinterpret_47/1
  in45/1 -> reinterpret_46/1
  in44/1 -> reinterpret_45/1
  in43/1 -> reinterpret_44/1
  in42/1 -> reinterpret_43/1
  in41/1 -> reinterpret_42/1
  in40/1 -> reinterpret_41/1
  in39/1 -> reinterpret_40/1
  in38/1 -> reinterpret_39/1
  in37/1 -> reinterpret_38/1
  in36/1 -> reinterpret_37/1
  in35/1 -> reinterpret_36/1
  in34/1 -> reinterpret_35/1
  in33/1 -> reinterpret_34/1
  in32/1 -> reinterpret_33/1
  in31/1 -> reinterpret_32/1
  in30/1 -> reinterpret_31/1
  in29/1 -> reinterpret_30/1
  in28/1 -> reinterpret_29/1
  in27/1 -> reinterpret_28/1
  in26/1 -> reinterpret_27/1
  in25/1 -> reinterpret_26/1
  in24/1 -> reinterpret_25/1
  in23/1 -> reinterpret_24/1
  in22/1 -> reinterpret_23/1
  in21/1 -> reinterpret_22/1
  in20/1 -> reinterpret_21/1
  in19/1 -> reinterpret_20/1
  in18/1 -> reinterpret_19/1
  in17/1 -> reinterpret_18/1
  in16/1 -> reinterpret_17/1
  in15/1 -> reinterpret_16/1
  in14/1 -> reinterpret_15/1
  in13/1 -> reinterpret_14/1
  in12/1 -> reinterpret_13/1
  in11/1 -> reinterpret_12/1
  in10/1 -> reinterpret_11/1
  in9/1 -> reinterpret_10/1
  in8/1 -> reinterpret_9/1
  in7/1 -> reinterpret_8/1
  in6/1 -> reinterpret_7/1
  in5/1 -> reinterpret_6/1
  in4/1 -> reinterpret_5/1
  in3/1 -> reinterpret_4/1
  in2/1 -> reinterpret_3/1
  in1/1 -> reinterpret_2/1
  in0/1 -> reinterpret_1/1

== system v01_aa_rii_16r4t11f_ver122sa/pfb_fir_real2/multi_delay_bram_fast_0/cram_all ==
  in0 : Inport
  in1 : Inport  Port=2
  in2 : Inport  Port=3
  in3 : Inport  Port=4
  in4 : Inport  Port=5
  in5 : Inport  Port=6
  in6 : Inport  Port=7
  in7 : Inport  Port=8
  in8 : Inport  Port=9
  in9 : Inport  Port=10
  in10 : Inport  Port=11
  concat : xbsIndex_r4/Concat  num_inputs=11  dbl_ovrd=off  block_type=concat
  reinterpret_{1..11} (11 alike) : xbsIndex_r4/Reinterpret  force_arith_type=on  arith_type=Unsigned  force_bin_pt=on  bin_pt=0  block_type=reinterpret
  out : Outport
  wires:
  concat/1 -> out/1
  reinterpret_11/1 -> concat/11
  reinterpret_10/1 -> concat/10
  reinterpret_9/1 -> concat/9
  reinterpret_8/1 -> concat/8
  reinterpret_7/1 -> concat/7
  reinterpret_6/1 -> concat/6
  reinterpret_5/1 -> concat/5
  reinterpret_4/1 -> concat/4
  reinterpret_3/1 -> concat/3
  reinterpret_2/1 -> concat/2
  reinterpret_1/1 -> concat/1
  in10/1 -> reinterpret_11/1
  in9/1 -> reinterpret_10/1
  in8/1 -> reinterpret_9/1
  in7/1 -> reinterpret_8/1
  in6/1 -> reinterpret_7/1
  in5/1 -> reinterpret_6/1
  in4/1 -> reinterpret_5/1
  in3/1 -> reinterpret_4/1
  in2/1 -> reinterpret_3/1
  in1/1 -> reinterpret_2/1
  in0/1 -> reinterpret_1/1
